simulink model "ofdm_txrx_supermimo_coded"  (Simulink 7.1)
settings: Solver=VariableStepDiscrete  SolverMode=SingleTasking  StartTime=0.0  StopTime=2*simOnly_simLength  RelTol=1e-3
PostLoadFcn:
  | ofdm_rx_supermimo_init
InitFcn:
  | ofdm_rx_supermimo_init
StartFcn:
  | ofdm_rx_supermimo_init
values from neighbouring files (symbols this model uses):
  Action0_Config = 0* 31*2^18 + 0*2^24 + PHY_AUTORESPONSE_REQ_GOODHDR + PHY_AUTORESPONSE_REQ_GOODPKT + PHY_AUTORESPONSE_REQ_FLAGA    [ofdm_tx_supermimo_init.m]
  Action1_Config = 0* 62*2^18 + PHY_AUTORESPONSE_REQ_GOODHDR + PHY_AUTORESPONSE_REQ_GOODPKT    [ofdm_tx_supermimo_init.m]
  Action2_Config = 0    [ofdm_tx_supermimo_init.m]
  Action3_Config = 0    [ofdm_tx_supermimo_init.m]
  Action4_Config = 0    [ofdm_tx_supermimo_init.m]
  Action5_Config = 0    [ofdm_tx_supermimo_init.m]
  ActionID_AFTransmit = 31    [ofdm_tx_supermimo_init.m]
  ActionID_Disabled = 0    [ofdm_tx_supermimo_init.m]
  ActionID_SetFlagA = 62    [ofdm_tx_supermimo_init.m]
  ActionID_SetFlagB = 61    [ofdm_tx_supermimo_init.m]
  BER_RAM_init_size = 2048    [ofdm_tx_supermimo_init.m]
  channel_h = [1 0; 1 0] * 0.3    [ofdm_tx_supermimo_init.m]
  CPLength = 16    [ofdm_tx_supermimo_init.m]
  FEC_Config = fec_coding_en*1 + fec_soft_dec*2 + fec_qpsk_scl*2^4 + fec_16qam_scl*2^8    (= 4195)    [ofdm_tx_supermimo_init.m]
  longCorr_coef_i = [-4*real(LTS) 0]    [ofdm_rx_supermimo_init.m]
  longCorr_coef_q = [4*imag(LTS) 0]    [ofdm_rx_supermimo_init.m]
  Match0_Config = 0    [ofdm_tx_supermimo_init.m]
  Match1_Config = 0    [ofdm_tx_supermimo_init.m]
  Match2_Config = 0    [ofdm_tx_supermimo_init.m]
  Match3_Config = 0    [ofdm_tx_supermimo_init.m]
  Match4_Config = 0    [ofdm_tx_supermimo_init.m]
  Match5_Config = 0    [ofdm_tx_supermimo_init.m]
  Match6_Config = 0    [ofdm_tx_supermimo_init.m]
  Match7_Config = 0    [ofdm_tx_supermimo_init.m]
  max_CP_length = 16    [ofdm_tx_supermimo_init.m]
  max_num_baseRateSymbols = 31    [ofdm_tx_supermimo_init.m]
  max_num_subcarriers = 64    [ofdm_tx_supermimo_init.m]
  max_num_trainingSymbols = 15    [ofdm_tx_supermimo_init.m]
  max_numBytes = 16384    [ofdm_tx_supermimo_init.m]
  max_OFDM_symbols = 1023    [ofdm_tx_supermimo_init.m]
  modConstellation_qam16 = .75*[1 3 -1 -3]./3    [ofdm_tx_supermimo_init.m]
  modConstellation_qam64 = 0.875*[3 1 5 7 -3 -1 -5 -7]./7    [ofdm_tx_supermimo_init.m]
  modConstellation_qpsk = [1 -1]./sqrt(2)    [ofdm_tx_supermimo_init.m]
  numBaseRateSymbols = numBaseRateSymbols *2    [ofdm_tx_supermimo_init.m]
  numSubcarriers = 64    [ofdm_tx_supermimo_init.m]
  numTrainingSymbols = 2    [ofdm_tx_supermimo_init.m]
  pilot_indicies = 7 + ( (64-21) * 2^8) + ( (64-7) * 2^16) + (21 * 2^24)    (= 356068103)    [ofdm_tx_supermimo_init.m]
  pilotValues = hex2dec('A57D') + (2^16 * hex2dec('5A82'))    [ofdm_tx_supermimo_init.m]
  pktByteNums = numHeaderBytes +  				byteIndex_numPayloadBytes(1)*2^8 +  				byteIndex_numPayloadBytes(2)*2^16 +  				byteIndex_simpleDynModMasks * 2^24    [ofdm_rx_supermimo_init.m]
  PktDet_Delay = 58+32 +      Symbol_Timing_Offset*2^7 +      0*2^16 +      (117* 2^24)    (= 1962935642)    [ofdm_rx_supermimo_init.m]
  pktTiming_count_payloadStart = pktTiming_count_lsStart + pktTiming_ls_num*pktTiming_ls_length    [ofdm_rx_supermimo_init.m]
  preamble = preamble_scale*[shortsyms_10 longsyms_2]    [ofdm_tx_supermimo_init.m]
  preamble_ROM = preamble_I_ROM + preamble_Q_ROM*2^16    [ofdm_tx_supermimo_init.m]
  RAM_init_size = max_numBytes    (= 16384)    [ofdm_tx_supermimo_init.m]
  reg_AF_Tx_Blanking = (320+80) + ( (320+80+79)*2^16)    (= 31392144)    [ofdm_rx_supermimo_init.m]
  reg_AF_Tx_Scaling = 2944    [ofdm_rx_supermimo_init.m]
  reg_Interrupt_PktBuf_Control = reg_InterruptControl +  	1 * 2^16 +  	0 * 2^24    (= 65536)    [ofdm_rx_supermimo_init.m]
  reg_PilotCalcParams = (4 * 2^16) + (minPilotChanMag)    (= 262184.96)    [ofdm_rx_supermimo_init.m]
  regRx_chanEst_minMag = ((0.5)*2^15) + ((0.5 * 2^15)*2^16)    (= 1073758208)    [ofdm_rx_supermimo_init.m]
  regRx_coarseCalcCorrection = round((1.3e-3 - 1.2982e-3)*2^32)    [ofdm_rx_supermimo_init.m]
  regRx_pilotCalcCorrection = round(1.00115 * 2^31)    [ofdm_rx_supermimo_init.m]
  regRx_preCFOoptions = 0 * 2^0 +  	1 * 2^1 +  	0    (= 2)    [ofdm_rx_supermimo_init.m]
  rx_controlBits = 1 * 2  		 + 1 * 4  		 + 0 * 8  		 + 1 * 16 * rx_SISO_Mode  		 + 1 * 32  		 + 1 * 64  		 + 1 * 128  		 + 1 * 256  		 + 1 * 512  		 + 1 * 1024  		 + 1 * 4096  		 + 0 * 16384  		 + 0 * 32768  		 + 1 * 65536  		 + 0 * 131072  		 + 0 * 262144  		 + 1 * 524288  		 + 1 * 1048576 * tx_Alamouti_Mode  		 + 0 * 2097152           + 1 * 33554432  		 + 0 * 4194304  		 + 0 * 8388608  		 + 0 * 16777216  		 + 1 *  ...(+49 chars)    [ofdm_rx_supermimo_init.m]
  Rx_PktDet_LongCorr_Params = LongCorrCounterLoad + 0*2^8 + 0*2^16 + 255*2^24    (= 4278190325)    [ofdm_rx_supermimo_init.m]
  Rx_PktDet_LongCorrThresholds = 8000    [ofdm_rx_supermimo_init.m]
  rx_postEq_scaling = round(rxScaling*2^11) * (1 + 2^16)    [ofdm_rx_supermimo_init.m]
  RxDataScrambling_Seq = [40 198 78 63 82 173 102 245 48 111 172 115 147 230 216 93 72 65 62 2 205 242 122 90 128 83 105 97 73 10 5 252]    [ofdm_tx_supermimo_init.m]
  RxReg_FixedPktLen = 0    [ofdm_rx_supermimo_init.m]
  rxReg_pktDetCorr = 2^00 * (floor(0.7*128)) +  	2^08 * (300) +  	0    [ofdm_rx_supermimo_init.m]
  shortSymbol_time = shortSymbol_time(1:16).'    [ofdm_tx_supermimo_init.m]
  simOnly_numSamples = length(preamble)+( (numSubcarriers+CPLength)*(numTrainingSymbols + numBaseRateSymbols + numFullRateSymbols/2) )    [ofdm_tx_supermimo_init.m]
  train = [train train]    [ofdm_tx_supermimo_init.m]
  tx_controlBits = (antB_preambleShift * 2^4) + (2^1 * tx_Alamouti_Mode) +  	(2^0 * tx_SISO_Mode) + 4*0 + 8*1 + 0*256 + 512*0 + 1024*0 +  	0*hex2dec('1000') + 0*hex2dec('2000') + 0*hex2dec('4000') +  	1*hex2dec('8000') + 1*hex2dec('10000') + hex2dec('20000') +  	0*hex2dec('100000')    [ofdm_tx_supermimo_init.m]
  tx_delays = (50*2^0) + (0*2^8) + (30*2^12)    (= 122930)    [ofdm_tx_supermimo_init.m]
  tx_scaling = (3072) + (12288 * 2^16)    (= 805309440)    [ofdm_tx_supermimo_init.m]
  TxDataScrambling_Seq = [40 198 78 63 82 173 102 245 48 111 172 115 147 230 216 93 72 65 62 2 205 242 122 90 128 83 105 97 73 10 5 252]    [ofdm_tx_supermimo_init.m]
  txReg_symbolCounts = (2^8 *numBaseRateSymbols ) + numTrainingSymbols    [ofdm_tx_supermimo_init.m]
  TxRx_FFTScaling = (3 * 2^0) +  	(2 * 2^2) +  	(1 * 2^4) +  	(1 * 2^6) +  	(1 * 2^8) +  	(0 * 2^10)    (= 347)    [ofdm_tx_supermimo_init.m]
undefined symbols (not defined in the file or in its folder): AntA, AntB, BaseRate, Down, gcbh, numBuffs, numBytes, Up, xlBlockLoadCallback, xlGetSimulinkPeriod, xlipgetpartsetting
libraries: xbsIndex_r4 x6000, simulink x4, dspsigops x4, commchan3 x2, dspsrcs4 x2, commrflib2 x2
(part 4 of 63)

== system ofdm_txrx_supermimo_coded/Memory-mapped Registers/AutoReply Registers ==
  Down Sample{1..14} (14 alike) : xbsIndex_r4/Down Sample  sample_ratio=2  sample_phase="Last Value of Frame  (most efficient)"  en=off  latency=1  dbl_ovrd=off  block_type=dsamp
      settings: block_version=10.1.3
  Read-Write Register1 : Subsystem  AncestorBlock=|  mask{regName='TxRx_AutoReply_Match1', out_dataType=Unsigned, out_numBits=32, out_binaryPt=0, initValue=Match1_Config, sampPeriod=1}
      | WARP_Blockset/Register Wrappers
      | (Sysgen 10.1+)/Read-Write Register
      mask prompts: regName "Register Name"; out_dataType "Output Type"; out_numBits "Output Num Bits"; out_binaryPt "Output Binary Point"; initValue "Initial Value"; sampPeriod "Sample Period"
      mask help:
      | This block is a wrapper for two registers from the System Generator Shared Memory blockset:
      | a) A "From Register" block, which provides a write-only register for the host processor
      | b) A "To Register" block, which provides a read-only register for the host processor
      |
      | Note: The Regisger Name paramter below must be unique for every instance of shared-memory registers in your system. System Generator will throw an error if duplicate names are found.
      mask init:
      | wo_regBlock = find_system(gcb, 'LookUnderMasks', 'all', 'FollowLinks', 'on', 'block_type', 'fromreg');
      | ro_regBlock = find_system(gcb, 'LookUnderMasks', 'all', 'FollowLinks', 'on', 'block_type', 'toreg');
      | 
      | if(length(wo_regBlock)>0)
      | 
      | 	%Set the shared memory name
      | 	set_param(wo_regBlock{1}, 'shared_memory_name', ['''' strcat(regName(2:end-1), '_w') '''']);
      | 
      | 	set_param(wo_regBlock{1}, 'arith_type', sprintf('%s', out_dataType));
      | 	set_param(wo_regBlock{1}, 'ownership', 'Locally owned and initialized')
      | 
      | 	set_param(wo_regBlock{1}, 'n_bits', num2str(out_numBits));
      | 	set_param(wo_regBlock{1}, 'bin_pt', num2str(out_binaryPt));
      | 	set_param(wo_regBlock{1}, 'init', num2str(initValue));
      | end
      | 
      | if(length(ro_regBlock)>0)
      | 
      | 	%Set the shared memory name
      | 	set_param(ro_regBlock{1}, 'shared_memory_name', ['''' strcat(regName(2:end-1), '_r') '''']);
      | 
      | 	set_param(ro_regBlock{1}, 'explicit_data_type', 'on')
      |     set_param(ro_regBlock{1}, 'arith_type', sprintf('%s', out_dataType));
      | 	set_param(ro_regBlock{1}, 'ownership', 'Locally owned and initialized')
      | 
      | 	set_param(ro_regBlock{1}, 'n_bits', num2str(out_numBits));
      | 	set_param(ro_regBlock{1}, 'bin_pt', num2str(out_binaryPt));
      | 	set_param(ro_regBlock{1}, 'init', num2str(initValue));
      | end
      settings: AttributesFormatString=RW Reg: %<regName>
      mask StyleString: edit,popup(Unsigned|Signed  (2's comp)),edit,edit,edit,edit
  Read-Write Register10 : Subsystem  AncestorBlock=|  mask{regName='TxRx_AutoReply_Action3', out_dataType=Unsigned, out_numBits=32, out_binaryPt=0, initValue=Action3_Config, sampPeriod=1}
      | WARP_Blockset/Register Wrappers
      | (Sysgen 10.1+)/Read-Write Register
      mask prompts: regName "Register Name"; out_dataType "Output Type"; out_numBits "Output Num Bits"; out_binaryPt "Output Binary Point"; initValue "Initial Value"; sampPeriod "Sample Period"
      mask help:
      | This block is a wrapper for two registers from the System Generator Shared Memory blockset:
      | a) A "From Register" block, which provides a write-only register for the host processor
      | b) A "To Register" block, which provides a read-only register for the host processor
      |
      | Note: The Regisger Name paramter below must be unique for every instance of shared-memory registers in your system. System Generator will throw an error if duplicate names are found.
      mask init: same as ofdm_txrx_supermimo_coded/Memory-mapped Registers/AutoReply Registers/Read-Write Register1
      settings: AttributesFormatString=RW Reg: %<regName>
      mask StyleString: edit,popup(Unsigned|Signed  (2's comp)),edit,edit,edit,edit
  Read-Write Register11 : Subsystem  AncestorBlock=|  mask{regName='TxRx_AutoReply_Action5', out_dataType=Unsigned, out_numBits=32, out_binaryPt=0, initValue=Action5_Config, sampPeriod=1}
      | WARP_Blockset/Register Wrappers
      | (Sysgen 10.1+)/Read-Write Register
      mask prompts: regName "Register Name"; out_dataType "Output Type"; out_numBits "Output Num Bits"; out_binaryPt "Output Binary Point"; initValue "Initial Value"; sampPeriod "Sample Period"
      mask help:
      | This block is a wrapper for two registers from the System Generator Shared Memory blockset:
      | a) A "From Register" block, which provides a write-only register for the host processor
      | b) A "To Register" block, which provides a read-only register for the host processor
      |
      | Note: The Regisger Name paramter below must be unique for every instance of shared-memory registers in your system. System Generator will throw an error if duplicate names are found.
      mask init: same as ofdm_txrx_supermimo_coded/Memory-mapped Registers/AutoReply Registers/Read-Write Register1
      settings: AttributesFormatString=RW Reg: %<regName>
      mask StyleString: edit,popup(Unsigned|Signed  (2's comp)),edit,edit,edit,edit
  Read-Write Register12 : Subsystem  AncestorBlock=|  mask{regName='TxRx_AutoReply_Action4', out_dataType=Unsigned, out_numBits=32, out_binaryPt=0, initValue=Action4_Config, sampPeriod=1}
      | WARP_Blockset/Register Wrappers
      | (Sysgen 10.1+)/Read-Write Register
      mask prompts: regName "Register Name"; out_dataType "Output Type"; out_numBits "Output Num Bits"; out_binaryPt "Output Binary Point"; initValue "Initial Value"; sampPeriod "Sample Period"
      mask help:
      | This block is a wrapper for two registers from the System Generator Shared Memory blockset:
      | a) A "From Register" block, which provides a write-only register for the host processor
      | b) A "To Register" block, which provides a read-only register for the host processor
      |
      | Note: The Regisger Name paramter below must be unique for every instance of shared-memory registers in your system. System Generator will throw an error if duplicate names are found.
      mask init: same as ofdm_txrx_supermimo_coded/Memory-mapped Registers/AutoReply Registers/Read-Write Register1
      settings: AttributesFormatString=RW Reg: %<regName>
      mask StyleString: edit,popup(Unsigned|Signed  (2's comp)),edit,edit,edit,edit
  Read-Write Register13 : Subsystem  AncestorBlock=|  mask{regName='TxRx_AutoReply_Match6', out_dataType=Unsigned, out_numBits=32, out_binaryPt=0, initValue=Match6_Config, sampPeriod=1}
      | WARP_Blockset/Register Wrappers
      | (Sysgen 10.1+)/Read-Write Register
      mask prompts: regName "Register Name"; out_dataType "Output Type"; out_numBits "Output Num Bits"; out_binaryPt "Output Binary Point"; initValue "Initial Value"; sampPeriod "Sample Period"
      mask help:
      | This block is a wrapper for two registers from the System Generator Shared Memory blockset:
      | a) A "From Register" block, which provides a write-only register for the host processor
      | b) A "To Register" block, which provides a read-only register for the host processor
      |
      | Note: The Regisger Name paramter below must be unique for every instance of shared-memory registers in your system. System Generator will throw an error if duplicate names are found.
      mask init: same as ofdm_txrx_supermimo_coded/Memory-mapped Registers/AutoReply Registers/Read-Write Register1
      settings: AttributesFormatString=RW Reg: %<regName>
      mask StyleString: edit,popup(Unsigned|Signed  (2's comp)),edit,edit,edit,edit
  Read-Write Register14 : Subsystem  AncestorBlock=|  mask{regName='TxRx_AutoReply_Match7', out_dataType=Unsigned, out_numBits=32, out_binaryPt=0, initValue=Match7_Config, sampPeriod=1}
      | WARP_Blockset/Register Wrappers
      | (Sysgen 10.1+)/Read-Write Register
      mask prompts: regName "Register Name"; out_dataType "Output Type"; out_numBits "Output Num Bits"; out_binaryPt "Output Binary Point"; initValue "Initial Value"; sampPeriod "Sample Period"
      mask help:
      | This block is a wrapper for two registers from the System Generator Shared Memory blockset:
      | a) A "From Register" block, which provides a write-only register for the host processor
      | b) A "To Register" block, which provides a read-only register for the host processor
      |
      | Note: The Regisger Name paramter below must be unique for every instance of shared-memory registers in your system. System Generator will throw an error if duplicate names are found.
      mask init: same as ofdm_txrx_supermimo_coded/Memory-mapped Registers/AutoReply Registers/Read-Write Register1
      settings: AttributesFormatString=RW Reg: %<regName>
      mask StyleString: edit,popup(Unsigned|Signed  (2's comp)),edit,edit,edit,edit
  Read-Write Register2 : Subsystem  AncestorBlock=|  mask{regName='TxRx_AutoReply_Match2', out_dataType=Unsigned, out_numBits=32, out_binaryPt=0, initValue=Match2_Config, sampPeriod=1}
      | WARP_Blockset/Register Wrappers
      | (Sysgen 10.1+)/Read-Write Register
      mask prompts: regName "Register Name"; out_dataType "Output Type"; out_numBits "Output Num Bits"; out_binaryPt "Output Binary Point"; initValue "Initial Value"; sampPeriod "Sample Period"
      mask help:
      | This block is a wrapper for two registers from the System Generator Shared Memory blockset:
      | a) A "From Register" block, which provides a write-only register for the host processor
      | b) A "To Register" block, which provides a read-only register for the host processor
      |
      | Note: The Regisger Name paramter below must be unique for every instance of shared-memory registers in your system. System Generator will throw an error if duplicate names are found.
      mask init: same as ofdm_txrx_supermimo_coded/Memory-mapped Registers/AutoReply Registers/Read-Write Register1
      settings: AttributesFormatString=RW Reg: %<regName>
      mask StyleString: edit,popup(Unsigned|Signed  (2's comp)),edit,edit,edit,edit
  Read-Write Register3 : Subsystem  AncestorBlock=|  mask{regName='TxRx_AutoReply_Match4', out_dataType=Unsigned, out_numBits=32, out_binaryPt=0, initValue=Match4_Config, sampPeriod=1}
      | WARP_Blockset/Register Wrappers
      | (Sysgen 10.1+)/Read-Write Register
      mask prompts: regName "Register Name"; out_dataType "Output Type"; out_numBits "Output Num Bits"; out_binaryPt "Output Binary Point"; initValue "Initial Value"; sampPeriod "Sample Period"
      mask help:
      | This block is a wrapper for two registers from the System Generator Shared Memory blockset:
      | a) A "From Register" block, which provides a write-only register for the host processor
      | b) A "To Register" block, which provides a read-only register for the host processor
      |
      | Note: The Regisger Name paramter below must be unique for every instance of shared-memory registers in your system. System Generator will throw an error if duplicate names are found.
      mask init: same as ofdm_txrx_supermimo_coded/Memory-mapped Registers/AutoReply Registers/Read-Write Register1
      settings: AttributesFormatString=RW Reg: %<regName>
      mask StyleString: edit,popup(Unsigned|Signed  (2's comp)),edit,edit,edit,edit
  Read-Write Register4 : Subsystem  AncestorBlock=|  mask{regName='TxRx_AutoReply_Match5', out_dataType=Unsigned, out_numBits=32, out_binaryPt=0, initValue=Match5_Config, sampPeriod=1}
      | WARP_Blockset/Register Wrappers
      | (Sysgen 10.1+)/Read-Write Register
      mask prompts: regName "Register Name"; out_dataType "Output Type"; out_numBits "Output Num Bits"; out_binaryPt "Output Binary Point"; initValue "Initial Value"; sampPeriod "Sample Period"
      mask help:
      | This block is a wrapper for two registers from the System Generator Shared Memory blockset:
      | a) A "From Register" block, which provides a write-only register for the host processor
      | b) A "To Register" block, which provides a read-only register for the host processor
      |
      | Note: The Regisger Name paramter below must be unique for every instance of shared-memory registers in your system. System Generator will throw an error if duplicate names are found.
      mask init: same as ofdm_txrx_supermimo_coded/Memory-mapped Registers/AutoReply Registers/Read-Write Register1
      settings: AttributesFormatString=RW Reg: %<regName>
      mask StyleString: edit,popup(Unsigned|Signed  (2's comp)),edit,edit,edit,edit
  Read-Write Register5 : Subsystem  AncestorBlock=|  mask{regName='TxRx_AutoReply_Match0', out_dataType=Unsigned, out_numBits=32, out_binaryPt=0, initValue=Match0_Config, sampPeriod=1}
      | WARP_Blockset/Register Wrappers
      | (Sysgen 10.1+)/Read-Write Register
      mask prompts: regName "Register Name"; out_dataType "Output Type"; out_numBits "Output Num Bits"; out_binaryPt "Output Binary Point"; initValue "Initial Value"; sampPeriod "Sample Period"
      mask help:
      | This block is a wrapper for two registers from the System Generator Shared Memory blockset:
      | a) A "From Register" block, which provides a write-only register for the host processor
      | b) A "To Register" block, which provides a read-only register for the host processor
      |
      | Note: The Regisger Name paramter below must be unique for every instance of shared-memory registers in your system. System Generator will throw an error if duplicate names are found.
      mask init: same as ofdm_txrx_supermimo_coded/Memory-mapped Registers/AutoReply Registers/Read-Write Register1
      settings: AttributesFormatString=RW Reg: %<regName>
      mask StyleString: edit,popup(Unsigned|Signed  (2's comp)),edit,edit,edit,edit
  Read-Write Register6 : Subsystem  AncestorBlock=|  mask{regName='TxRx_AutoReply_Match3', out_dataType=Unsigned, out_numBits=32, out_binaryPt=0, initValue=Match3_Config, sampPeriod=1}
      | WARP_Blockset/Register Wrappers
      | (Sysgen 10.1+)/Read-Write Register
      mask prompts: regName "Register Name"; out_dataType "Output Type"; out_numBits "Output Num Bits"; out_binaryPt "Output Binary Point"; initValue "Initial Value"; sampPeriod "Sample Period"
      mask help:
      | This block is a wrapper for two registers from the System Generator Shared Memory blockset:
      | a) A "From Register" block, which provides a write-only register for the host processor
      | b) A "To Register" block, which provides a read-only register for the host processor
      |
      | Note: The Regisger Name paramter below must be unique for every instance of shared-memory registers in your system. System Generator will throw an error if duplicate names are found.
      mask init: same as ofdm_txrx_supermimo_coded/Memory-mapped Registers/AutoReply Registers/Read-Write Register1
      settings: AttributesFormatString=RW Reg: %<regName>
      mask StyleString: edit,popup(Unsigned|Signed  (2's comp)),edit,edit,edit,edit
  Read-Write Register7 : Subsystem  AncestorBlock=|  mask{regName='TxRx_AutoReply_Action0', out_dataType=Unsigned, out_numBits=32, out_binaryPt=0, initValue=Action0_Config, sampPeriod=1}
      | WARP_Blockset/Register Wrappers
      | (Sysgen 10.1+)/Read-Write Register
      mask prompts: regName "Register Name"; out_dataType "Output Type"; out_numBits "Output Num Bits"; out_binaryPt "Output Binary Point"; initValue "Initial Value"; sampPeriod "Sample Period"
      mask help:
      | This block is a wrapper for two registers from the System Generator Shared Memory blockset:
      | a) A "From Register" block, which provides a write-only register for the host processor
      | b) A "To Register" block, which provides a read-only register for the host processor
      |
      | Note: The Regisger Name paramter below must be unique for every instance of shared-memory registers in your system. System Generator will throw an error if duplicate names are found.
      mask init: same as ofdm_txrx_supermimo_coded/Memory-mapped Registers/AutoReply Registers/Read-Write Register1
      settings: AttributesFormatString=RW Reg: %<regName>
      mask StyleString: edit,popup(Unsigned|Signed  (2's comp)),edit,edit,edit,edit
  Read-Write Register8 : Subsystem  AncestorBlock=|  mask{regName='TxRx_AutoReply_Action1', out_dataType=Unsigned, out_numBits=32, out_binaryPt=0, initValue=Action1_Config, sampPeriod=1}
      | WARP_Blockset/Register Wrappers
      | (Sysgen 10.1+)/Read-Write Register
      mask prompts: regName "Register Name"; out_dataType "Output Type"; out_numBits "Output Num Bits"; out_binaryPt "Output Binary Point"; initValue "Initial Value"; sampPeriod "Sample Period"
      mask help:
      | This block is a wrapper for two registers from the System Generator Shared Memory blockset:
      | a) A "From Register" block, which provides a write-only register for the host processor
      | b) A "To Register" block, which provides a read-only register for the host processor
      |
      | Note: The Regisger Name paramter below must be unique for every instance of shared-memory registers in your system. System Generator will throw an error if duplicate names are found.
      mask init: same as ofdm_txrx_supermimo_coded/Memory-mapped Registers/AutoReply Registers/Read-Write Register1
      settings: AttributesFormatString=RW Reg: %<regName>
      mask StyleString: edit,popup(Unsigned|Signed  (2's comp)),edit,edit,edit,edit
  Read-Write Register9 : Subsystem  AncestorBlock=|  mask{regName='TxRx_AutoReply_Action2', out_dataType=Unsigned, out_numBits=32, out_binaryPt=0, initValue=Action2_Config, sampPeriod=1}
      | WARP_Blockset/Register Wrappers
      | (Sysgen 10.1+)/Read-Write Register
      mask prompts: regName "Register Name"; out_dataType "Output Type"; out_numBits "Output Num Bits"; out_binaryPt "Output Binary Point"; initValue "Initial Value"; sampPeriod "Sample Period"
      mask help:
      | This block is a wrapper for two registers from the System Generator Shared Memory blockset:
      | a) A "From Register" block, which provides a write-only register for the host processor
      | b) A "To Register" block, which provides a read-only register for the host processor
      |
      | Note: The Regisger Name paramter below must be unique for every instance of shared-memory registers in your system. System Generator will throw an error if duplicate names are found.
      mask init: same as ofdm_txrx_supermimo_coded/Memory-mapped Registers/AutoReply Registers/Read-Write Register1
      settings: AttributesFormatString=RW Reg: %<regName>
      mask StyleString: edit,popup(Unsigned|Signed  (2's comp)),edit,edit,edit,edit
  tag scope: regAutoReply_action0=global, regAutoReply_action1=global, regAutoReply_action2=global, regAutoReply_action3=global, regAutoReply_action4=global, regAutoReply_action5=global, regAutoReply_match0=global, regAutoReply_match1=global, regAutoReply_match2=global, regAutoReply_match3=global, regAutoReply_match4=global, regAutoReply_match5=global, regAutoReply_match6=global, regAutoReply_match7=global
  wires:
  Read-Write Register5/1(D) -> Down Sample1/1
  Read-Write Register1/1(D) -> Down Sample2/1
  Read-Write Register2/1(D) -> Down Sample3/1
  Read-Write Register6/1(D) -> Down Sample4/1
  Read-Write Register3/1(D) -> Down Sample5/1
  Read-Write Register4/1(D) -> Down Sample6/1
  Read-Write Register7/1(D) -> Down Sample12/1
  Read-Write Register8/1(D) -> Down Sample11/1
  Read-Write Register9/1(D) -> Down Sample10/1
  Read-Write Register10/1(D) -> Down Sample9/1
  Read-Write Register12/1(D) -> Down Sample8/1
  Read-Write Register11/1(D) -> Down Sample7/1
  Down Sample1/1 -> [regAutoReply_match0]
  Down Sample2/1 -> [regAutoReply_match1]
  Down Sample3/1 -> [regAutoReply_match2]
  Down Sample4/1 -> [regAutoReply_match3]
  Down Sample5/1 -> [regAutoReply_match4]
  Down Sample6/1 -> [regAutoReply_match5]
  Down Sample7/1 -> [regAutoReply_action5]
  Down Sample8/1 -> [regAutoReply_action4]
  Down Sample9/1 -> [regAutoReply_action3]
  Down Sample10/1 -> [regAutoReply_action2]
  Down Sample11/1 -> [regAutoReply_action1]
  Down Sample12/1 -> [regAutoReply_action0]
  Read-Write Register13/1(D) -> Down Sample13/1
  Down Sample13/1 -> [regAutoReply_match6]
  Read-Write Register14/1(D) -> Down Sample14/1
  Down Sample14/1 -> [regAutoReply_match7]
